simulink model "FluxModel2015a_verify_Q_zhixin"  (Simulink 10.2) (continued)
== system FluxModel2015a_verify_Q_zhixin/Note == (continued)
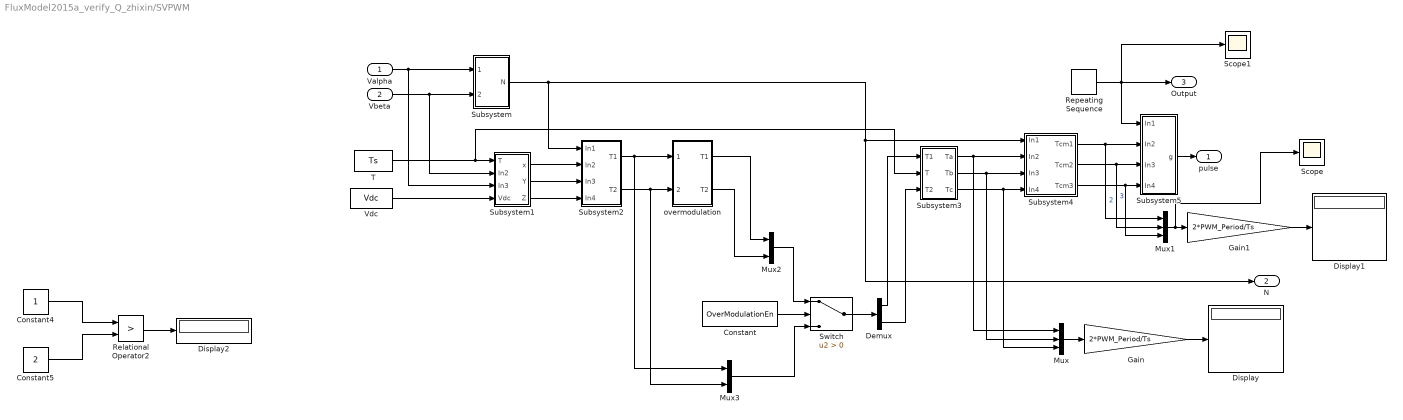
== system FluxModel2015a_verify_Q_zhixin/SVPWM ==
  Valpha : Inport
  Vbeta : Inport  Port=2
  Constant : Constant  Value=OverModulationEn
  Constant4 : Constant
  Constant5 : Constant  Value=2
  Demux : Demux  Outputs=2
  Display : Display
      settings: Decimation=2
  Display1 : Display
      settings: Decimation=2
  Display2 : Display
  Gain : Gain  Gain=2*PWM_Period/Ts
  Gain1 : Gain  Gain=2*PWM_Period/Ts
  Mux : Mux  Inputs=3
  Mux1 : Mux  Inputs=3
  Mux2 : Mux  Inputs=2
  Mux3 : Mux  Inputs=2
  Relational Operator2 : RelationalOperator  Operator=>  OutDataTypeStr=boolean  RndMeth=Simplest
  Repeating Sequence : simulink/Sources/Repeating Sequence  rep_seq_t=[0 Ts/2 Ts]  rep_seq_y=[0 Ts/2 0]
  Scope : Scope
  Scope1 : Scope
  Subsystem : Subsystem
  Subsystem1 : Subsystem
  Subsystem2 : Subsystem
  Subsystem3 : Subsystem
  Subsystem4 : Subsystem
  Subsystem5 : Subsystem
  Switch : Switch  Criteria=u2 > Threshold
  T : Constant  Value=Ts
  Vdc : Constant  Value=Vdc
  overmodulation : Subsystem
  pulse : Outport
  N : Outport  Port=2
  Output : Outport  Port=3
  wires:
  Subsystem5/1(g) -> pulse/1
  Repeating Sequence/1 -> Scope1/1, Subsystem5/1(In1), Output/1
  Subsystem4/3(Tcm3) -> Mux1/3, Subsystem5/4(In4)
  Subsystem4/2(Tcm2) -> Mux1/2, Subsystem5/3(In3)  "3"
  Subsystem4/1(Tcm1) -> Mux1/1, Subsystem5/2(In2)  "2"
  Subsystem3/3(Tc) -> Subsystem4/4(In4), Mux/3
  Subsystem3/2(Tb) -> Subsystem4/3(In3), Mux/2
  Subsystem3/1(Ta) -> Subsystem4/2(In2), Mux/1
  Subsystem1/3(Z) -> Subsystem2/4(In4)
  Subsystem1/2(Y) -> Subsystem2/3(In3)
  Subsystem1/1(x) -> Subsystem2/2(In2)
  Subsystem/1(N) -> Subsystem4/1(In1), N/1, Subsystem2/1(In1)
  Vdc/1 -> Subsystem1/4(Vdc)
  T/1 -> Subsystem3/2(T), Subsystem1/1(T)
  Vbeta/1 -> Subsystem1/2(In2), Subsystem/2(Vbeta)
  Valpha/1 -> Subsystem1/3(In3), Subsystem/1(Valpha)
  Mux/1 -> Gain/1
  Gain/1 -> Display/1
  Mux1/1 -> Scope/1, Gain1/1
  Gain1/1 -> Display1/1
  Constant4/1 -> Relational Operator2/1
  Constant5/1 -> Relational Operator2/2
  Relational Operator2/1 -> Display2/1
  Subsystem2/1(T1) -> Mux3/1, overmodulation/1(1)
  Subsystem2/2(T2) -> overmodulation/2(2), Mux3/2
  Constant/1 -> Switch/2
  overmodulation/1(T1) -> Mux2/1
  overmodulation/2(T2) -> Mux2/2
  Mux2/1 -> Switch/1
  Mux3/1 -> Switch/3
  Switch/1 -> Demux/1
  Demux/1 -> Subsystem3/1(T1)
  Demux/2 -> Subsystem3/3(T2)

== system FluxModel2015a_verify_Q_zhixin/Signal Builder ==
  Demux : Demux  Tag=STV Demux  Outputs=1
      settings: DisplayOption=none
  FromWs : FromWorkspace  Tag=STV FromWs  VariableName=tuvar  SigBuilderData=DataTag1  VnvData=DataTag2
      SigBuilderData=|
      | common.afterFinalStr = 'Extrapolate'
      | common.sampleTime = 0
      | dataSet.timeRange = [0 10]
      | dataSet.name = 'Group 1'
      | sbobj.Groups.Name = 'Group 1'
      | sbobj.Groups.Signals.Name = 'Signal 2'
      | sbobj.Groups.Signals.XData = [0 5 5 10]
      | sbobj.Groups.Signals.YData = [0 1 1 0]
  Signal 2 : Outport  Tag=STV Outport
  wires:
  FromWs/1 -> Demux/1
  Demux/1 -> Signal 2/1

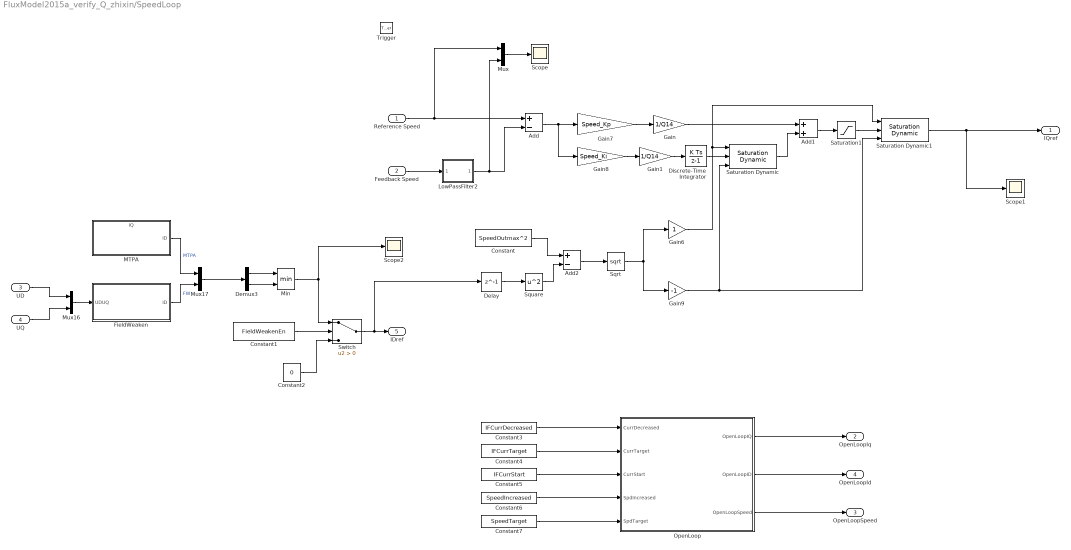
== system FluxModel2015a_verify_Q_zhixin/SpeedLoop ==
  Reference Speed : Inport
  Feedback Speed : Inport  Port=2
  UD : Inport  Port=3
  UQ : Inport  Port=4
  Trigger : TriggerPort  VariantControl=Variant  PortDimensions=1
  Add : Sum  Inputs=+-
  Add1 : Sum
  Add2 : Sum  Inputs=+-
  Constant : Constant  Value=SpeedOutmax^2
  Constant1 : Constant  Value=FieldWeakenEn
  Constant2 : Constant  Value=0
  Constant3 : Constant  Value=IFCurrDecreased
  Constant4 : Constant  Value=IFCurrTarget
  Constant5 : Constant  Value=IFCurrStart
  Constant6 : Constant  Value=SpeedIncreased
  Constant7 : Constant  Value=SpeedTarget
  Delay : Delay  DelayLength=1
  Demux3 : Demux  Outputs=2
  Discrete-Time Integrator : DiscreteIntegrator  gainval=1.0/tb  SampleTime=-1  LimitOutput=on  UpperSaturationLimit=SpeedOutmax  LowerSaturationLimit=-SpeedOutmax  ICPrevOutput=DiscIntNeverNeededParam  ICPrevScaledInput=DiscIntNeverNeededParam
  FieldWeaken : Subsystem
  Gain : Gain  Gain=1/Q14
  Gain1 : Gain  Gain=1/Q14
  Gain6 : Gain
  Gain7 : Gain  Gain=Speed_Kp
  Gain8 : Gain  Gain=Speed_Ki
  Gain9 : Gain  Gain=-1
  LowPassFilter2 : Subsystem
  MTPA : Subsystem
      settings: PortSchema="{\"entries\":[{\"content\":{\"sides\":[{\"content\":{\"connectorIds\":[]},\"type\":\"ConnectorPlacement.EquallySpacedRectSide\",\"uuid\":\"a5904245-4ea6-4d6d-a524-a9289c0225a6\"},{\"content\":{\"connectorIds\":[\"Out1\"],\"side\":\"RIGHT\"},\"type\":\"ConnectorPlacement.EquallySpacedRectSide\",\"uuid\":\"60983ff3-a156-4868-be5e-bf62d665d4eb\"},{\"content\":{\"connectorIds\":[\"In1\"],\"side\":\"TOP\"},\"type\":\"ConnectorPlacement.EquallySpacedRectSide\",\"uuid\":\"474b3f1b-69f7-4d3b-95eb-2704c9a64f09\"},{\"content\":{\"connectorIds\":[],\"side\":\"HIDDEN\"},\"type\":\"ConnectorPlacement.EquallySpacedRectSide\",\"uuid\":\"4d5a81f1-6675-4864-8749-0d48e39305a2\"}]},\"type\":\"ConnectorPlacement.EquallySpacedConnector\",\"uuid\":\"566d7f91-92d4-4d90-8232-38b94aeb0503\"}],\"packageUris\":[\"http://schema.mathworks.com/mf0/Connector/R2020b\"],\"version\":\"1.0\"}"
  Min : MinMax  Inputs=2
  Mux : Mux  Inputs=2
  Mux16 : Mux  Inputs=2
  Mux17 : Mux  Inputs=2
  OpenLoop : Subsystem
  Saturation Dynamic : simulink/Discontinuities/Saturation Dynamic  OutMin=[]  OutMax=[]  OutDataTypeStr=Inherit: Same as second input  OutDataType=fixdt(1, 16)  OutScaling=2^-10  DoSatur=off
  Saturation Dynamic1 : simulink/Discontinuities/Saturation Dynamic  OutMin=[]  OutMax=[]  OutDataTypeStr=Inherit: Same as second input  OutDataType=fixdt(1, 16)  OutScaling=2^-10  DoSatur=off
  Saturation1 : Saturate  UpperLimit=SpeedOutmax  LowerLimit=-SpeedOutmax
  Scope : Scope
  Scope1 : Scope
  Scope2 : Scope
  Sqrt : Sqrt  SaturateOnIntegerOverflow=on
  Square : Math  Operator=square  SaturateOnIntegerOverflow=on
  Switch : Switch  Criteria=u2 > Threshold
  IQref : Outport
  OpenLoopIq : Outport  Port=2
  OpenLoopSpeed : Outport  Port=3
  OpenLoopId : Outport  Port=4
  IDref : Outport  Port=5
  wires:
  Add/1 -> Gain7/1, Gain8/1
  Gain9/1 -> Saturation Dynamic1/3, Saturation Dynamic/3
  Discrete-Time Integrator/1 -> Saturation Dynamic/2
  FieldWeaken/1(ID) -> Mux17/2  "FW"
  Add2/1 -> Sqrt/1
  Square/1 -> Add2/2
  Gain6/1 -> Saturation Dynamic1/1, Saturation Dynamic/1
  Delay/1 -> Square/1
  Demux3/2 -> Min/2
  Add1/1 -> Saturation1/1
  UD/1 -> Mux16/1
  Saturation Dynamic/1 -> Add1/2
  UQ/1 -> Mux16/2
  Constant/1 -> Add2/1
  Sqrt/1 -> Gain9/1, Gain6/1
  Mux16/1 -> FieldWeaken/1(UDUQ)
  Mux17/1 -> Demux3/1
  MTPA/1(ID) -> Mux17/1  "MTPA"
  Reference Speed/1 -> Mux/1, Add/1
  Demux3/1 -> Min/1
  OpenLoop/1(OpenLoopIQ) -> OpenLoopIq/1
  Constant3/1 -> OpenLoop/1(CurrDecreased)
  Constant5/1 -> OpenLoop/3(CurrStart)
  OpenLoop/3(OpenLoopSpeed) -> OpenLoopSpeed/1
  Constant7/1 -> OpenLoop/5(SpdTarget)
  Constant6/1 -> OpenLoop/4(SpdIncreased)
  Constant4/1 -> OpenLoop/2(CurrTarget)
  OpenLoop/2(OpenLoopID) -> OpenLoopId/1
  Mux/1 -> Scope/1
  Gain7/1 -> Gain/1
  Gain8/1 -> Gain1/1
  Min/1 -> Switch/1, Scope2/1
  Saturation Dynamic1/1 -> Scope1/1, IQref/1
  Saturation1/1 -> Saturation Dynamic1/2
  Gain/1 -> Add1/1
  Gain1/1 -> Discrete-Time Integrator/1
  Constant1/1 -> Switch/2
  Switch/1 -> Delay/1, IDref/1
  Constant2/1 -> Switch/3
  Feedback Speed/1 -> LowPassFilter2/1(Input)
  LowPassFilter2/1(Output) -> Mux/2, Add/2

== system FluxModel2015a_verify_Q_zhixin/CurrLoop&Observer/GivenAngle ==
  Speed : Inport
  Discrete-Time Integrator : DiscreteIntegrator  gainval=1.0/tb  SampleTime=-1  ICPrevOutput=DiscIntNeverNeededParam  ICPrevScaledInput=DiscIntNeverNeededParam
  Fcn1 : Fcn  Expr=rem((u(1)),(2*pi))
  Fcn2 : Fcn  Expr=rem((u(1)),(65535))
  Gain : Gain  Gain=10430
  Gain1 : Gain  Gain=(2*pi)/65535
  Mux : Mux  Inputs=3
  Mux1 : Mux  Inputs=2
  Scope : Scope
  Scope1 : Scope
  Angle : Outport
  wires:
  Fcn2/1 -> Mux1/1, Mux/2, Gain1/1
  Speed/1 -> Discrete-Time Integrator/1
  Discrete-Time Integrator/1 -> Fcn1/1, Gain/1
  Gain/1 -> Mux/3, Fcn2/1
  Gain1/1 -> Mux/1, Angle/1
  Mux/1 -> Scope/1
  Fcn1/1 -> Mux1/2
  Mux1/1 -> Scope1/1

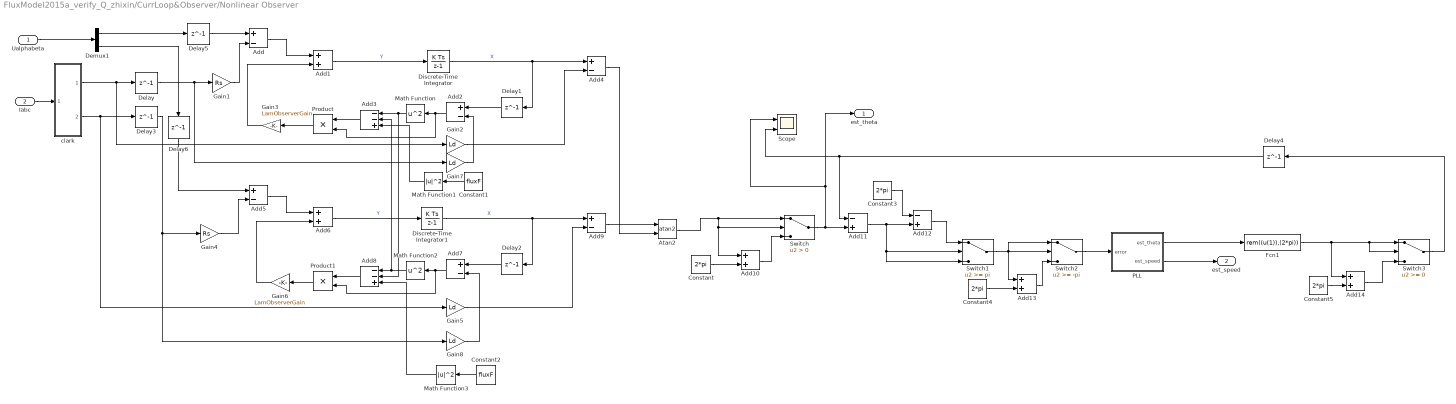
== system FluxModel2015a_verify_Q_zhixin/CurrLoop&Observer/Nonlinear Observer ==
  Ualphabeta : Inport
  Iabc : Inport  Port=2
  Add{,2,4..5,7,9} (6 alike) : Sum  Inputs=+-
  Add{1,6,10,13..14} (5 alike) : Sum
  Add11 : Sum  Inputs=-+
  Add12 : Sum  Inputs=-+
  Add3 : Sum  Inputs=--+
  Add8 : Sum  Inputs=--+
  Atan2 : Trigonometry  Operator=atan2
  Constant{,3..5} (4 alike) : Constant  Value=2*pi
  Constant1 : Constant  Value=fluxF
  Constant2 : Constant  Value=fluxF
  Delay{,1..6} (7 alike) : Delay  DelayLength=1
  Demux1 : Demux  Outputs=2
  Discrete-Time Integrator : DiscreteIntegrator  gainval=1.0/tb  InitialCondition=fluxF  SampleTime=-1  ICPrevOutput=DiscIntNeverNeededParam  ICPrevScaledInput=DiscIntNeverNeededParam
  Discrete-Time Integrator1 : DiscreteIntegrator  gainval=1.0/tb  SampleTime=-1  ICPrevOutput=DiscIntNeverNeededParam  ICPrevScaledInput=DiscIntNeverNeededParam
  Fcn1 : Fcn  Expr=rem((u(1)),(2*pi))
  Gain1 : Gain  Gain=Rs
  Gain{2,5,7..8} (4 alike) : Gain  Gain=Ld
  Gain3 : Gain  Gain=LamObserverGain
  Gain4 : Gain  Gain=Rs
  Gain6 : Gain  Gain=LamObserverGain
  Math Function : Math  Operator=square  SaturateOnIntegerOverflow=on
  Math Function1 : Math  Operator=magnitude^2  SaturateOnIntegerOverflow=on
  Math Function2 : Math  Operator=square  SaturateOnIntegerOverflow=on
  Math Function3 : Math  Operator=magnitude^2  SaturateOnIntegerOverflow=on
  PLL : Subsystem
  Product : Product
  Product1 : Product
  Scope : Scope  NumInputPorts=2
  Switch : Switch  Criteria=u2 > Threshold
  Switch1 : Switch  Threshold=pi
  Switch2 : Switch  Threshold=-pi
  Switch3 : Switch
  clark : Subsystem
  est_theta : Outport
  est_speed : Outport  Port=2
  wires:
  Constant2/1 -> Math Function3/1
  Math Function2/1 -> Add3/2, Add8/1
  Iabc/1 -> clark/1(Iabc)
  Add7/1 -> Math Function2/1, Product1/2
  Add1/1 -> Discrete-Time Integrator/1  "Y"
  Math Function3/1 -> Add8/3
  Demux1/2 -> Delay6/1
  Gain1/1 -> Add/2
  Delay2/1 -> Add7/1
  Math Function/1 -> Add3/1, Add8/2
  Discrete-Time Integrator1/1 -> Delay2/1, Add9/1  "X"
  Gain6/1 -> Add6/2
  Product1/1 -> Gain6/1
  Add8/1 -> Product1/1
  Demux1/1 -> Delay5/1
  Add2/1 -> Product/2, Math Function/1
  Add6/1 -> Discrete-Time Integrator1/1  "Y"
  Product/1 -> Gain3/1
  Delay1/1 -> Add2/1
  Discrete-Time Integrator/1 -> Add4/1, Delay1/1  "X"
  Add5/1 -> Add6/1
  Gain4/1 -> Add5/2
  clark/1(Ialpha) -> Gain2/1, Delay/1
  Add/1 -> Add1/1
  Gain2/1 -> Add4/2
  Constant1/1 -> Math Function1/1
  Gain3/1 -> Add1/2
  Math Function1/1 -> Add3/3
  clark/2(Ibeta) -> Delay3/1, Gain5/1
  Add3/1 -> Product/1
  Ualphabeta/1 -> Demux1/1
  Delay/1 -> Gain7/1, Gain1/1
  Delay3/1 -> Gain8/1, Gain4/1
  Add4/1 -> Atan2/2
  Add9/1 -> Atan2/1
  Atan2/1 -> Switch/1, Add10/1, Switch/2
  Add10/1 -> Switch/3
  Constant/1 -> Add10/2
  Switch/1 -> Scope/1, est_theta/1, Add11/2
  Add11/1 -> Switch1/3, Switch1/2, Add12/2
  Constant3/1 -> Add12/1
  Add12/1 -> Switch1/1
  Switch1/1 -> Switch2/1, Add13/1, Switch2/2
  Add13/1 -> Switch2/3
  Constant4/1 -> Add13/2
  Switch2/1 -> PLL/1(error)
  PLL/2(est_speed) -> est_speed/1
  PLL/1(est_theta) -> Fcn1/1
  Fcn1/1 -> Add14/1, Switch3/2, Switch3/1
  Add14/1 -> Switch3/3
  Constant5/1 -> Add14/2
  Switch3/1 -> Delay4/1
  Delay4/1 -> Add11/1, Scope/2
  Delay5/1 -> Add/1
  Delay6/1 -> Add5/1
  Gain5/1 -> Add9/2
  Gain7/1 -> Add2/2
  Gain8/1 -> Add7/2

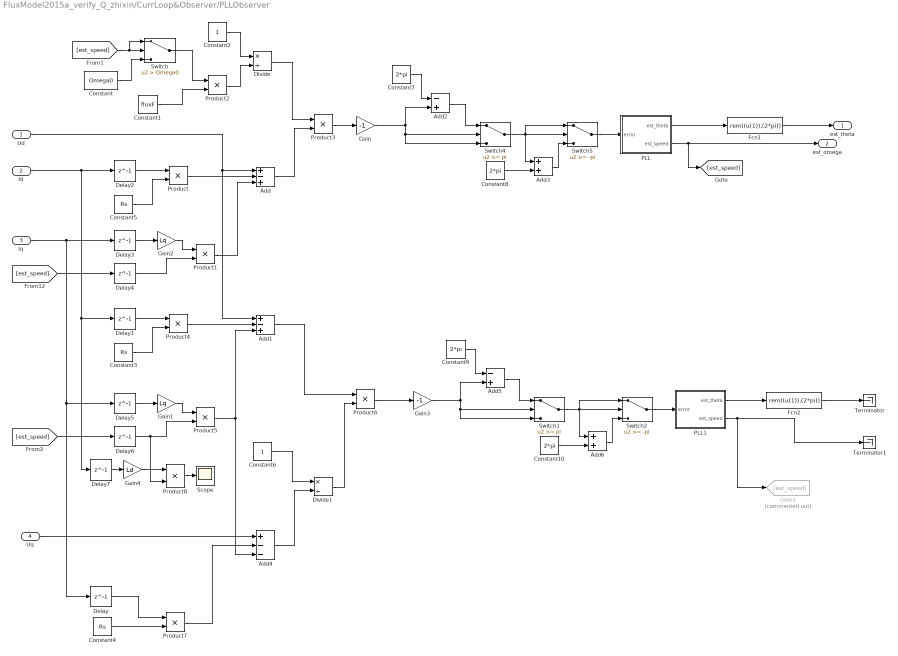
== system FluxModel2015a_verify_Q_zhixin/CurrLoop&Observer/PLLObserver ==
  Ud : Inport
  Id : Inport  Port=2
  Iq : Inport  Port=3
  Uq : Inport  Port=4
  Add : Sum  Inputs=+-+
  Add1 : Sum  Inputs=+-+
  Add2 : Sum  Inputs=-+
  Add3 : Sum
  Add4 : Sum  Inputs=+--
  Add5 : Sum  Inputs=-+
  Add6 : Sum
  Constant : Constant  Value=Omega0
  Constant1 : Constant  Value=fluxF
  Constant{7..10} (4 alike) : Constant  Value=2*pi
  Constant2 : Constant
  Constant3 : Constant  Value=Rs
  Constant4 : Constant  Value=Rs
  Constant5 : Constant  Value=Rs
  Constant6 : Constant
  Delay{,1..7} (8 alike) : Delay  DelayLength=1
  Divide : Product  Inputs=*/
  Divide1 : Product  Inputs=*/
  Fcn1 : Fcn  Expr=rem((u(1)),(2*pi))
  Fcn2 : Fcn  Expr=rem((u(1)),(2*pi))
  Gain : Gain  Gain=-1
  Gain1 : Gain  Gain=Lq
  Gain2 : Gain  Gain=Lq
  Gain3 : Gain  Gain=-1
  Gain4 : Gain  Gain=Ld
  Goto1 : Goto  Commented=on  GotoTag=est_speed
  PLL : Subsystem
  PLL1 : Subsystem
  Product{,1..8} (9 alike) : Product
  Scope : Scope
  Switch : Switch  Criteria=u2 > Threshold  Threshold=Omega0
  Switch1 : Switch  Threshold=pi
  Switch2 : Switch  Threshold=-pi
  Switch4 : Switch  Threshold=pi
  Switch5 : Switch  Threshold=-pi
  Terminator : Terminator
  Terminator1 : Terminator
  est_theta : Outport
  est_omega : Outport  Port=2
  wires:
  Constant5/1 -> Product/2
  [est_speed] -> Delay4/1
  Delay4/1 -> Product1/2
  Gain2/1 -> Product1/1
  Delay3/1 -> Gain2/1
  Product1/1 -> Add/3
  Product/1 -> Add/2
  Delay2/1 -> Product/1
  Id/1 -> Delay7/1, Delay1/1, Delay2/1
  Add/1 -> Product3/2
  [est_speed] -> Switch/1, Switch/2
  Constant/1 -> Switch/3
  Switch/1 -> Product2/1
  Constant1/1 -> Product2/2
  Constant2/1 -> Divide/1
  Product2/1 -> Divide/2
  Divide/1 -> Product3/1
  Switch4/1 -> Switch5/1, Add3/1, Switch5/2
  PLL/2(est_speed) -> est_omega/1, [est_speed]
  Add3/1 -> Switch5/3
  Constant8/1 -> Add3/2
  Constant7/1 -> Add2/1
  Add2/1 -> Switch4/1
  Switch5/1 -> PLL/1(error)
  Product3/1 -> Gain/1
  Gain/1 -> Switch4/3, Switch4/2, Add2/2
  PLL/1(est_theta) -> Fcn1/1
  Constant3/1 -> Product4/2
  Product4/1 -> Add1/2
  Delay1/1 -> Product4/1
  Ud/1 -> Add1/1, Add/1
  Delay6/1 -> Product8/2, Product5/2
  Gain1/1 -> Product5/1
  Delay5/1 -> Gain1/1
  Iq/1 -> Delay/1, Delay5/1, Delay3/1
  Product5/1 -> Add4/3, Add1/3
  Add1/1 -> Product6/1
  Uq/1 -> Add4/1
  Delay/1 -> Product7/1
  Constant4/1 -> Product7/2
  Product7/1 -> Add4/2
  Constant6/1 -> Divide1/1
  Add4/1 -> Divide1/2
  Divide1/1 -> Product6/2
  Product6/1 -> Gain3/1
  Switch1/1 -> Switch2/1, Add6/1, Switch2/2
  Constant9/1 -> Add5/1
  Constant10/1 -> Add6/2
  Switch2/1 -> PLL1/1(error)
  Add5/1 -> Switch1/1
  Gain3/1 -> Switch1/3, Switch1/2, Add5/2
  Add6/1 -> Switch2/3
  PLL1/1(est_theta) -> Fcn2/1
  Fcn2/1 -> Terminator/1
  PLL1/2(est_speed) -> Terminator1/1, Goto1/1
  Fcn1/1 -> est_theta/1
  Delay7/1 -> Gain4/1
  [est_speed] -> Delay6/1
  Gain4/1 -> Product8/1
  Product8/1 -> Scope/1

== system FluxModel2015a_verify_Q_zhixin/CurrLoop&Observer/Park ==
  alpha : Inport
  beta : Inport  Port=2
  theta : Inport  Port=3
  Add : Sum
  Cos : Trigonometry  Operator=cos
  Product{,1..3} (4 alike) : Product
  Sin : Trigonometry
  Subtract1 : Sum  Inputs=+-
  ID : Outport
  IQ : Outport  Port=2
  wires:
  Product2/1 -> Subtract1/1
  beta/1 -> Product2/1, Product/1
  theta/1 -> Cos/1, Sin/1
  Sin/1 -> Product3/2, Product/2
  alpha/1 -> Product3/1, Product1/2
  Cos/1 -> Product1/1, Product2/2
  Subtract1/1 -> IQ/1
  Product1/1 -> Add/2
  Product3/1 -> Subtract1/2
  Product/1 -> Add/1
  Add/1 -> ID/1

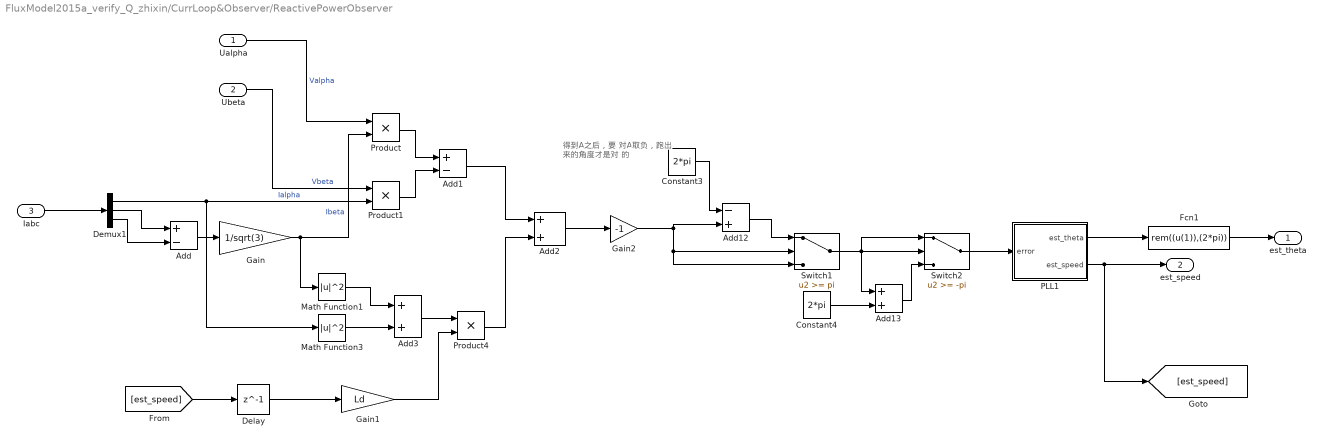
== system FluxModel2015a_verify_Q_zhixin/CurrLoop&Observer/ReactivePowerObserver ==
  note: 得到A之后，要
      | 对A取负，跑出
      | 来的角度才是对
      | 的
  Ualpha : Inport
  Ubeta : Inport  Port=2
  Iabc : Inport  Port=3
  Add : Sum  Inputs=+-
  Add1 : Sum  Inputs=+-
  Add12 : Sum  Inputs=-+
  Add13 : Sum
  Add2 : Sum
  Add3 : Sum
  Constant3 : Constant  Value=2*pi
  Constant4 : Constant  Value=2*pi
  Delay : Delay  DelayLength=1
  Demux1 : Demux  Outputs=3
  Fcn1 : Fcn  Expr=rem((u(1)),(2*pi))
  Gain : Gain  Gain=1/sqrt(3)
  Gain1 : Gain  Gain=Ld
  Gain2 : Gain  Gain=-1
  Math Function1 : Math  Operator=magnitude^2  SaturateOnIntegerOverflow=on
  Math Function3 : Math  Operator=magnitude^2  SaturateOnIntegerOverflow=on
  PLL1 : Subsystem
  Product : Product
  Product1 : Product
  Product4 : Product
  Switch1 : Switch  Threshold=pi
  Switch2 : Switch  Threshold=-pi
  est_theta : Outport
  est_speed : Outport  Port=2
  wires:
  Demux1/1 -> Product1/2, Math Function3/1  "Ialpha"
  Product1/1 -> Add1/2
  Math Function1/1 -> Add3/1
  Demux1/2 -> Add/1
  Gain/1 -> Product/2, Math Function1/1  "Ibeta"
  Add1/1 -> Add2/1
  Ubeta/1 -> Product1/1  "Vbeta"
  Ualpha/1 -> Product/1  "Valpha"
  Product/1 -> Add1/1
  Add/1 -> Gain/1
  Demux1/3 -> Add/2
  Product4/1 -> Add2/2
  Add3/1 -> Product4/1
  Gain1/1 -> Product4/2
  Iabc/1 -> Demux1/1
  Constant4/1 -> Add13/2
  Switch2/1 -> PLL1/1(error)
  Switch1/1 -> Switch2/1, Add13/1, Switch2/2
  PLL1/1(est_theta) -> Fcn1/1
  Constant3/1 -> Add12/1
  Add12/1 -> Switch1/1
  PLL1/2(est_speed) -> [est_speed], est_speed/1
  Add13/1 -> Switch2/3
  Math Function3/1 -> Add3/2
  Fcn1/1 -> est_theta/1
  [est_speed] -> Delay/1
  Delay/1 -> Gain1/1
  Add2/1 -> Gain2/1
  Gain2/1 -> Switch1/3, Switch1/2, Add12/2

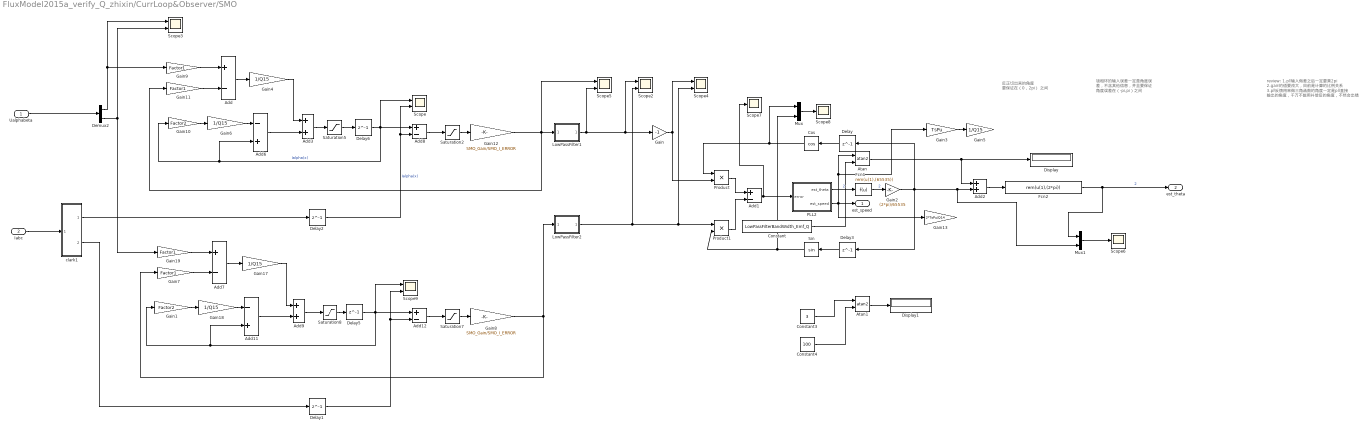
== system FluxModel2015a_verify_Q_zhixin/CurrLoop&Observer/SMO ==
  note: 反正切出来的角度
      | 要保证在（0，2pi）
      | 之间
  note: 锁相环的输入误差一定是角度误
      | 差，不含其他信息，并且要保证
      | 角度误差在（-pi,pi）之间
  note: review:
      | 1.pll输入做差之后一定要乘2pi
      | 2.gain的值要改大，目前是计算的比例关系
      | 3.pll反馈用来做三角函数的角度一定是pll直接
      | 输出的角度，千万不能用补偿后的角度，不然会出错
  Ualphabeta : Inport
  Iabc : Inport  Port=2
  Add{,1,7..8,12} (5 alike) : Sum  Inputs=+-
  Add11 : Sum  Inputs=-+
  Add2 : Sum
  Add3 : Sum
  Add6 : Sum  Inputs=-+
  Add9 : Sum
  Atan : Trigonometry  Operator=atan2
  Atan1 : Trigonometry  Operator=atan2
  Constant : Constant  Value=LowPassFilterBandWidth_Emf_Q
  Constant3 : Constant  Value=3
  Constant4 : Constant  Value=100
  Cos : Trigonometry  Operator=cos
  Delay{,1..3,5..6} (6 alike) : Delay  DelayLength=1
  Demux2 : Demux  Outputs=2
  Display : Display
  Display1 : Display
  Fcn1 : Fcn  Expr=rem(u(1),(65535))
  Fcn2 : Fcn  Expr=rem(u(1),(2*pi))
  Gain : Gain  Gain=-1
  Gain1 : Gain  Gain=Factor2
  Gain10 : Gain  Gain=Factor2
  Gain{7,9,11,19} (4 alike) : Gain  Gain=Factor1
  Gain12 : Gain  Gain=SMO_Gain/SMO_I_ERROR
  Gain13 : Gain  Gain=2*TsPu/Q14
  Gain{4..6,17..18} (5 alike) : Gain  Gain=1/Q15
  Gain2 : Gain  Gain=(2*pi)/65535
  Gain3 : Gain  Gain=TsPu
  Gain8 : Gain  Gain=SMO_Gain/SMO_I_ERROR
  LowPassFilter1 : Subsystem
  LowPassFilter2 : Subsystem
  Mux : Mux  Inputs=2
  Mux1 : Mux  Inputs=2
  PLL2 : Subsystem
  Product : Product
  Product1 : Product
  Saturation2 : Saturate  UpperLimit=SMO_I_ERROR  LowerLimit=-SMO_I_ERROR
  Saturation5 : Saturate  UpperLimit=16384  LowerLimit=-16384
  Saturation7 : Saturate  UpperLimit=SMO_I_ERROR  LowerLimit=-SMO_I_ERROR
  Saturation8 : Saturate  UpperLimit=16384  LowerLimit=-16384
  Scope{,2..5,9} (6 alike) : Scope  NumInputPorts=2
  Scope6 : Scope
  Scope7 : Scope
  Scope8 : Scope
  Sin : Trigonometry
  clark1 : Subsystem
  est_speed : Outport
  est_theta : Outport  Port=2
  wires:
  Demux2/1 -> Scope3/1, Gain9/1
  Gain9/1 -> Add/1
  Saturation2/1 -> Gain12/1
  Gain11/1 -> Add/2
  Add8/1 -> Saturation2/1
  Delay6/1 -> Scope/1, Add8/1, Add6/2, Gain10/1  "Ialpha(x)"
  Delay/1 -> Cos/1
  Cos/1 -> Mux/1, Product/1
  Delay3/1 -> Sin/1
  Sin/1 -> Mux/2, Product1/2
  Product/1 -> Add1/1
  Product1/1 -> Add1/2
  PLL2/2(est_speed) -> Gain13/1, Gain3/1, Atan/1, est_speed/1
  Add1/1 -> PLL2/1(error), Scope7/1
  PLL2/1(est_theta) -> Fcn1/1  "2"
  Constant/1 -> Atan/2
  Fcn2/1 -> Mux1/1, est_theta/1  "2"
  Add2/1 -> Fcn2/1
  Constant3/1 -> Atan1/1
  Constant4/1 -> Atan1/2
  Atan1/1 -> Display1/1
  LowPassFilter1/1(Output) -> Scope5/2, Scope2/1, Gain/1
  Add/1 -> Gain4/1
  Gain4/1 -> Add3/1
  Ualphabeta/1 -> Demux2/1
  Iabc/1 -> clark1/1(Iabc)
  Delay2/1 -> Scope/2, Add8/2  "Ialpha(x)"
  Gain/1 -> Product/2, Scope4/1
  LowPassFilter2/1(Output) -> Scope2/2, Product1/1, Scope4/2
  Mux/1 -> Scope8/1
  Atan/1 -> Add2/1, Display/1
  clark1/1(Ialpha) -> Delay2/1
  Gain12/1 -> Scope5/1, LowPassFilter1/1(Input), Gain11/1
  Add6/1 -> Add3/2
  Gain10/1 -> Gain6/1
  Gain6/1 -> Add6/1
  Add11/1 -> Add9/2
  Gain1/1 -> Gain18/1
  Gain18/1 -> Add11/1
  Gain7/1 -> Add7/2
  clark1/2(Ibeta) -> Delay1/1
  Gain8/1 -> LowPassFilter2/1(Input), Gain7/1
  Saturation7/1 -> Gain8/1
  Add7/1 -> Gain17/1
  Gain17/1 -> Add9/1
  Delay5/1 -> Scope9/1, Add12/1, Add11/2, Gain1/1
  Gain19/1 -> Add7/1
  Demux2/2 -> Gain19/1, Scope3/2
  Add12/1 -> Saturation7/1
  Delay1/1 -> Scope9/2, Add12/2
  Add3/1 -> Saturation5/1
  Saturation5/1 -> Delay6/1
  Add9/1 -> Saturation8/1
  Saturation8/1 -> Delay5/1
  Fcn1/1 -> Gain2/1  "2"
  Mux1/1 -> Scope6/1
  Gain3/1 -> Gain5/1
  Gain2/1 -> Mux1/2, Add2/2, Delay3/1, Delay/1

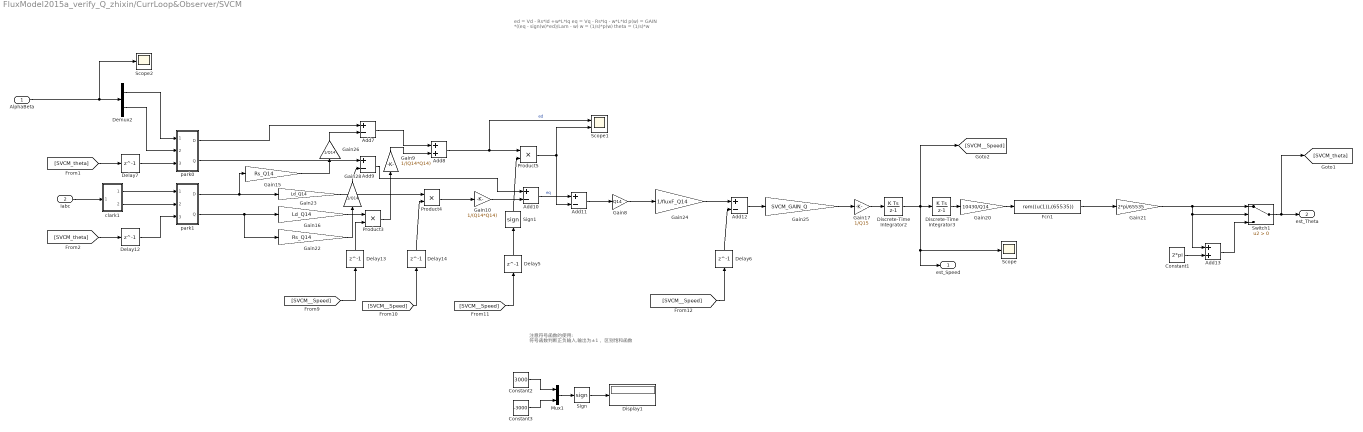
== system FluxModel2015a_verify_Q_zhixin/CurrLoop&Observer/SVCM ==
  note: 注意符号函数的使用:
      | 符号函数判断正负输入,输出为±1，
      | 区别饱和函数
  note: ed = Vd - Rs*Id +w*L*Iq
      | eq = Vq - Rs*Iq - w*L*Id
      | p(w) = GAIN *((eq - sign(w)*ed)/Lam - w)
      | w = (1/s)*p(w)
      | theta = (1/s)*w
  AlphaBeta : Inport
  Iabc : Inport  Port=2
  Add{7,9..12} (5 alike) : Sum  Inputs=+-
  Add13 : Sum
  Add8 : Sum
  Constant1 : Constant  Value=2*pi
  Constant2 : Constant  Value=3000
  Constant3 : Constant  Value=-3000
  Delay{5..7,12..14} (6 alike) : Delay  DelayLength=1
  Demux2 : Demux  Outputs=2
  Discrete-Time Integrator2 : DiscreteIntegrator  gainval=1.0/tb  SampleTime=-1  LimitOutput=on  UpperSaturationLimit=EstOutMax_Speed_Q  LowerSaturationLimit=-EstOutMax_Speed_Q  ICPrevOutput=DiscIntNeverNeededParam  ICPrevScaledInput=DiscIntNeverNeededParam
  Discrete-Time Integrator3 : DiscreteIntegrator  gainval=1.0/tb  SampleTime=-1  UpperSaturationLimit=EstOutMax_Theta  LowerSaturationLimit=-EstOutMax_Theta  ICPrevOutput=DiscIntNeverNeededParam  ICPrevScaledInput=DiscIntNeverNeededParam
  Display1 : Display
      settings: Decimation=2
  Fcn1 : Fcn  Expr=rem((u(1)),(65535))
  Gain10 : Gain  Gain=1/(Q14*Q14)
  Gain15 : Gain  Gain=Rs_Q14
  Gain16 : Gain  Gain=Ld_Q14
  Gain17 : Gain  Gain=1/Q15
  Gain20 : Gain  Gain=10430/Q14
  Gain21 : Gain  Gain=2*pi/65535
  Gain22 : Gain  Gain=Rs_Q14
  Gain23 : Gain  Gain=Ld_Q14
  Gain24 : Gain  Gain=1/fluxF_Q14
  Gain25 : Gain  Gain=SVCM_GAIN_Q
  Gain26 : Gain  Gain=1/Q14
  Gain28 : Gain  Gain=1/Q14
  Gain8 : Gain  Gain=Q14
  Gain9 : Gain  Gain=1/(Q14*Q14)
  Mux1 : Mux  Inputs=2
  Product3 : Product
  Product4 : Product
  Product5 : Product
  Scope : Scope
  Scope1 : Scope  NumInputPorts=2
  Scope2 : Scope
  Sign : Signum
  Sign1 : Signum
  Switch1 : Switch  Criteria=u2 > Threshold
  clark1 : Subsystem
  park0 : Subsystem
  park1 : Subsystem
  est_Speed : Outport
  est_Theta : Outport  Port=2
  wires:
  Gain25/1 -> Gain17/1
  Discrete-Time Integrator3/1 -> Gain20/1
  Product3/1 -> Gain9/1
  Gain26/1 -> Add7/2
  Gain28/1 -> Add9/2
  Switch1/1 -> est_Theta/1, [SVCM_theta]
  Delay14/1 -> Product4/2
  Add13/1 -> Switch1/3
  Demux2/1 -> park0/1(alpha)
  Demux2/2 -> park0/2(beta)
  Delay7/1 -> park0/3(theta)
  Delay12/1 -> park1/3(theta)
  [SVCM_theta] -> Delay7/1
  Gain15/1 -> Gain26/1
  Add11/1 -> Gain8/1
  clark1/2(Ibeta) -> park1/2(beta)
  [SVCM__Speed] -> Delay6/1
  clark1/1(Ialpha) -> park1/1(alpha)
  Product4/1 -> Gain10/1
  Gain22/1 -> Gain28/1
  Add12/1 -> Gain25/1
  Add7/1 -> Add8/1
  Add10/1 -> Add11/1  "eq"
  Add8/1 -> Scope1/1, Product5/1  "ed"
  Constant1/1 -> Add13/2
  [SVCM_theta] -> Delay12/1
  Gain24/1 -> Add12/1
  Gain16/1 -> Product3/1
  Delay6/1 -> Add12/2
  Add9/1 -> Add10/1
  Gain23/1 -> Product4/1
  Delay13/1 -> Product3/2
  Iabc/1 -> clark1/1(Iabc)
  Fcn1/1 -> Gain21/1
  Gain20/1 -> Fcn1/1
  Gain21/1 -> Switch1/1, Add13/1, Switch1/2
  Discrete-Time Integrator2/1 -> Scope/1, est_Speed/1, [SVCM__Speed], Discrete-Time Integrator3/1
  Gain9/1 -> Add8/2
  Gain10/1 -> Add10/2
  [SVCM__Speed] -> Delay13/1
  [SVCM__Speed] -> Delay14/1
  [SVCM__Speed] -> Delay5/1
  Product5/1 -> Add11/2, Scope1/2
  Sign1/1 -> Product5/2
  Delay5/1 -> Sign1/1
  Gain8/1 -> Gain24/1
  Gain17/1 -> Discrete-Time Integrator2/1
  Sign/1 -> Display1/1
  Constant2/1 -> Mux1/1
  Constant3/1 -> Mux1/2
  Mux1/1 -> Sign/1
  AlphaBeta/1 -> Scope2/1, Demux2/1
  park0/1(D) -> Add7/1
  park0/2(Q) -> Add9/1
  park1/1(D) -> Gain23/1, Gain15/1
  park1/2(Q) -> Gain22/1, Gain16/1
